simulink model "HDVSim_FCEV_Truck"  (R2019b)
settings: Solver=ode23s  StartTime=0.0  StopTime=inf  FixedStep=sample_time  MaxStep=0.1  RelTol=10e-1  EnableMultiTasking=on
model workspace (MAT data in the model file):
  Anschaffungskosten = [3707764736; 2; 1; 1; 1; 1]
  Bat.Useable_range = 0.1
  Bat.Useable_capacity = 87.5
  Bat.Type = 2
  Bat.SOC_start = 0.5
  Bat.Voltage = 800
  Bat.Efficiency_power_electronics = 0.95
  Bat.Charge_cycles = 3000
  DrvTrn = 14
  Eigenschaften = [3707764736; 2; 1; 1; 2; 2]
  Electric_Truck = 1
  Elektro_LKW = 1
  Factor_EM = 0.2
  Fahrzyklus = 2
  FuelCell.P_nom = 120
  FuelCell.NernstVoltage = [1121.27882197 1115.6396755]
  FuelCell.OperatingPoint = [171.428571429 700]
  FuelCell.MaximumPoint = [244.897959184 625.010310793]
  FuelCell.NoCells = 1015
  FuelCell.Eta = 55
  FuelCell.OperatingTemp = 65
  FuelCell.AirFlow = 7145.36039252
  FuelCell.Pressure = [1.5 1]
  FuelCell.Composition = [99.95 21 1]
  FuelCell.regV = [700 748.201780912 802.997904409 873.900542152 1076.08341487]
  FuelCell.regI = [171.428571429 128.571428571 85.7142857143 42.8571428571 2]
  FuelCell.regulation1 = [171.428571429 700]
  FuelCell.regulation2 = [128.571428571 748.201780912]
  FuelCell.regulation3 = [85.7142857143 802.997904409]
  FuelCell.regulation4 = [42.8571428571 873.900542152]
  FuelCell.regulation5 = [2 1076.08341487]
  FuelCell.Hysterese1 = [0.35 0.3]
  FuelCell.Hysterese2 = [0.4 0.35]
  FuelCell.Hysterese3 = [0.45 0.4]
  FuelCell.Hysterese4 = [0.5 0.45]
  FuelCell.Hysterese5 = [0.55 0.5]
  Fueltype = 13
  Gasart = ''
  Gewichte = [3707764736; 2; 1; 1; 3; 3]
  Hybrid_LKW = 1
  Hybrid_Truck = 1
  IZ12 = 3600
  Kosten.Kges = 1.08061767244
  Kosten.Kges_oP = 0.797760529586
  Kosten.KA = 0.104662721893
  Kosten.KA_ZM = 49440
  Kosten.KA_AN1 = 39000
  Kosten.KA_AN2 = 0
  Kosten.KA_AN3 = 0
  Kosten.tab = 6.5
  Kosten.sj = 130000
  Kosten.KK = 0.46991
  Kosten.KK_Diesel = 1.3426
  Kosten.BK_Diesel = 35
  Kosten.KK_CNG = 1.094
  Kosten.BK_CNG = 0
  Kosten.KK_LNG = 0.5671
  Kosten.BK_LNG = 0
  Kosten.IK = 100
  Kosten.KSS = 0.0046991
  Kosten.f_SS = 0.01
  Kosten.KAB = 0.0187964
  Kosten.f_AB = 0.04
  Kosten.KR = 0.0323076923077
  Kosten.KR_ZM = 400
  Kosten.BR_ZM = 6
  Kosten.KR_AN = 300
  Kosten.BR_AN = 6
  Kosten.IR = 130000
  Kosten.KW = 0.0230769230769
  Kosten.KW_ZM = 1500
  Kosten.BW = 2
  Kosten.IW = 130000
  Kosten.KV = 0.0384615384615
  Kosten.KV_ZM = 5000
  Kosten.IV = 130000
  Kosten.KM = 0.0987
  Kosten.KM_ZM = 0.141
  Kosten.f_M = 0.7
  Kosten.KS = 0.00714615384615
  Kosten.KS_ZM = 556
  Kosten.KS_AN = 373
  Kosten.IS = 130000
  Kosten.KP = 0.282857142857
  Kosten.KPSL = 19.8
  Kosten.v_avg = 70
  Kraftstoff = 13
  M_el = 1000
  Opt_groessen = []
  Parallel = 'no'
  SOC_T_EM_completely_available = 0.5
  SOC_addition = 0.4
  SOC_el = 0.8
  SOC_soll = 0.5
  SOC_target = 0.85
  Sailing_without_drag_torque = 1
  TCO = []
  TCO_Anhaenger = 1
  TCO_Trailer = 1
  T_distance_LPS_down = 250
  T_distance_LPS_up = 250
  Temp1 = [1000 1100 1200 1300 1400 1500 1600 1700 1800 1900 2000 2100 2200 2300 2400 2500 2600 2700 2800 2900 3000 3100 3200 3300 3400 3500 3600 3700 3800 3900 4000 4100 4200 4300 4400 4500 4600 4700 4800 4900 5000]
  VSim.Display = 2
  VSim.Opt = 0
  VSim.v_t = [0 0 0 0 0 0 0 0 0 0 0 0 0.1 2.22673669893 4.45336306907 5.92398131548 10.3757847916 13.2235931536 16.4384727928 19.3208442034 20.4098221432 22.5036755202 24.4402958137 25.2224117846 25.2414500209 26.5896480968 27.764074406 29.7317382984 29.2489710153 29.8958446635 31.3306398255 32.4945605422 32.2484373969 32.7472665177 34.574070202 36.2762220764 37.8657378187 37.7612251955 37.2633218769 37.960097988 39.1551886552 40.0333329677 41.4355684859 42.768336292 44.0386638817 45.25244579 46.4146842031 46.9654264036 46.4489997818 46.5754869226 47.2481025657 48.3417532766 49.3936383063 50.4067716597 51.3838125461 52.0475410751 52.7867950368 53.6833902649 54.5511282108 55.3916308392 56.206370499 56.9966877803 57.6121852605 58.3615642383 59.0897061106 59.7975811134 60.4860862919 61.1560531125 61.8082544664 62.4434104654 63.0621929387 63.6652304544 64.2531124249 64.8263914568 65.3855880065 65.9311920811 66.4636658567 66.9834460991 67.4909462072 67.9865577959 68.4706523474 68.94358275 69.4056845665 69.8572774365 70.2986658222 70.730140154 71.1519781486 71.5644446766 71.9677937776 72.3622683873 72.7481013003 73.1255161942 73.4947274795 73.8559412098 74.2093555521 74.555161059 74.8935412643 75.22467292 75.5487264183 75.86586614 76.1762506856 76.4800736479 76.7776508183 77.0691489472 77.354700525 77.6344340882 77.9084742343 78.1769412151 78.4399520593 78.6976199742 78.9500548666 79.1483067825 79.3915890977 79.6299292459 79.863425809 80.004951896 80.18236505 80.4046218047 80.6223491669 80.8356342124 81.0445617915 81.2492142762 81.4496719512 81.6460130853 81.8383140741 82.0266493037]
  Vehicle = 'FCEV'
  WPT.Voltage = 0
  WPT.P_max = 200
  WPT.expansion = 0.3
  WPT.eta = 0.92
  WPT.SOC_target = 0.8
  WPT.SOC_electric_only = 0.3
  acquisitionCosts = []
  acquisition_costs = [3707764736; 2; 1; 1; 3; 3]
  addition_for_critical_slope = 0.01
  ambient.gravity = 9.80665
  ambient.air_density = 1.1883
  ambient.friction_coeff = 0.8
  auxiliary_consumers.onBattery = 0
  auxiliary_consumers.power = 3500
  critical_altitude_difference = 50
  cycle.distance = [0 1 2 3 4 5 6 7 8 9 10 11 ... (92937 elements, 92937x1)]
  cycle.speed = [0 23.0555555556 23.0555555556 23.0555555556 23.0555555556 23.0555555556 23.0555555556 23.0555555556 23.0555555556 23.0555555556 23.0555555556 23.0555555556 ... (92937 elements, 92937x1)]
  cycle.slope = [-0.008925 -0.008925 -0.0089836957 -0.0090130435 -0.0090423913 -0.0090717391 -0.009101087 -0.0091304348 -0.0091597826 -0.0091891304 -0.0092184783 -0.0092478261 ... (92937 elements, 92937x1)]
  cycle.stop_time = [1 1 1 0 0 0 0 0 0 0 0 0 ... (92937 elements, 92937x1)]
  cycle.altitude = [0 0 0 0 0 0 0 0 0 0 0 0 ... (92937 elements, 92937x1)]
  cycle.stop_end = 15
  cycle.stop_start = 5
  cycle.speed_init = 1
  cycle.delta12 = [0 0 0 0 0 0 0 0 0 0 0 0 ... (92937 elements, 1x92937)]
  cycle.delta23 = [0 0 0 0 0 0 0 0 0 0 0 0 ... (92937 elements, 1x92937)]
  dcycle = 21
  distance_1_PPC = 175
  distance_2_PPC = 200
  distance_PPC_altitude_difference = 10000
  driver.controller_proportional_gain = 230000
  driver.controller_integral_gain = 3000
  driver.slew_rate_throttle_in = 2
  driver.slew_rate_throttle_out = -2
  driver.slew_rate_brake_in = 2
  driver.slew_rate_brake_out = -2
  em.M_max = 1600
  em.n_max = 10000
  em.n_eck = 2800
  em.P_max = 469.144502936
  em.shift_parameter.n_pref = 2632
  em.shift_parameter.n2 = 588
  em.shift_parameter.n6 = 8000
  em.shift_parameter.M_max = 1600
  em.fuel.co2_per_kwh = 478
  em.speed = [0 10 20 30 40 50 60 70 80 90 100 110 ... (1001 elements, 1x1001)]
  em.trq = [1600 1600 1600 1600 1600 1600 1600 1600 1600 1600 1600 1600 ... (1001 elements, 1x1001)]
  em.Type = 2
  em.efficiency.characteristic_map = [0.001 0.001 0.001 0.001 0.001 0.001 0.001 0.001 0.001 0.001 0.001 0.001 ... (20301 elements, 101x201)]
  em.efficiency.speed = [0 50 100 150 200 250 300 350 400 450 500 550 600 650 700 750 800 850 900 950 1000 1050 1100 1150 1200 1250 1300 1350 1400 1450 1500 1550 1600 1650 1700 1750 1800 1850 1900 1950 2000 2050 2100 2150 2200 2250 2300 2350 2400 2450 2500 2550 2600 2650 2700 2750 2800 2850 2900 2950 3000 3050 3100 3150 3200 3250 3300 3350 3400 3450 3500 3550 3600 3650 3700 3750 3800 3850 3900 3950 4000 4050 4100 4150 4200 4250 4300 4350 4400 4450 4500 4550 4600 4650 4700 4750 4800 4850 4900 4950 5000 5050 5100 5150 5200 5250 5300 5350 5400 5450 5500 5550 5600 5650 5700 5750 5800 5850 5900 5950 6000 6050 6100 6150 6200 6250 6300 6350 6400 6450 6500 6550 6600 6650 6700 6750 6800 6850 6900 6950 7000 7050 7100 7150 7200 7250 7300 7350 7400 7450 7500 7550 7600 7650 7700 7750 7800 7850 7900 7950 8000 8050 8100 8150 8200 8250 8300 8350 8400 8450 8500 8550 8600 8650 8700 8750 8800 8850 8900 8950 9000 9050 9100 9150 9200 9250 9300 9350 9400 9450 9500 9550 9600 9650 9700 9750 9800 9850 9900 9950 10000]
  em.efficiency.torque = [0 16 32 48 64 80 96 112 128 144 160 176 192 208 224 240 256 272 288 304 320 336 352 368 384 400 416 432 448 464 480 496 512 528 544 560 576 592 608 624 640 656 672 688 704 720 736 752 768 784 800 816 832 848 864 880 896 912 928 944 960 976 992 1008 1024 1040 1056 1072 1088 1104 1120 1136 1152 1168 1184 1200 1216 1232 1248 1264 1280 1296 1312 1328 1344 1360 1376 1392 1408 1424 1440 1456 1472 1488 1504 1520 1536 1552 1568 1584 1600]
  em.noEM = 2
  em_M_max = 1563
  engine.shift_parameter.n_lo = 1024
  engine.shift_parameter.n_pref = 1354
  engine.M_max = 0
  engine.number = 4
  final_drive.ratio = 10
  final_drive.trq_eff = 0.98
  ifElectric = 1
  ifOptimized = 1
  look_ahead_PPC = 250
  max_distance = 100185
  name = 'HDVSim_FCEV_Truck'
  preditiveCruiseControl = 1
  propertie = [3707764736; 2; 1; 1; 4; 4]
  prospective_cruise_control = 1
  sample_time = 0.1
  tank.v_diesel = 0
  tank.v_cng = 0
  tank.v_lng = 0
  tank.m_h2 = 20
  tires.radius = 0.4465
  tires.roll_drag_coeff.total = 0.007
  transmission.init_gear = 1
  transmission.shift_time = 1
  transmission.ratios = [3.0257 2.42471496127 1.94310164372 1.55714962713 1.24785801561 1]
  transmission.trq_eff = [0.96 0.966 0.972 0.978 0.984 0.99]
  transmission.lamda = [1.08679703675 1.05406616627 1.03254008792 1.02251301421 1.01826306417 1.0130058518]
  transmission.z = 6
  v_PPC_delta = 2.77777777778
  v_max_electrical_drive_only = 13.8888888889
  vehicle.long_truck = 0
  vehicle.payload = 19300
  vehicle.frontal_area = 10.2
  vehicle.air_drag_coeff = 0.53
  vehicle.Fx_max = 90221.18
  vehicle.F_brake_max = 309890.14
  vehicle.mass = 16370.7055705
  vehicleProperties = []
  vehicle_F_brake_max = 309890.14
  weights = []
  x = [1600 19 0.1 87.5 10 3.0257 4 0 0 1024 1354 2 2 120]
values from neighbouring files (symbols this model uses):
  FuelCell = FuelCell_design(180, 600)    [Parameterizing.m]
  transmission = Transmission_gearing(15.86, 7, 0, 0, final_drive.ratio)    [Parameterizing.m]
undefined symbols (not defined in the file or in its folder): Ion, Lithium, Vorausschauender_Tempomat
libraries: simulink x22, powerlib x4, electricdrivelib x2
(part 1 of 3)
settings Model: Creator=Alexander Suessmann  LastModifiedBy=ubuntu
settings SolverCC: ZeroCrossControl=EnableAll  AutoInsertRateTranBlk=on
settings DataIOCC: SaveFormat=Array  SignalLoggingSaveFormat=ModelDataLogs  SaveOutput=off  SignalLogging=off  DSMLogging=off  ReturnWorkspaceOutputs=off  SignalLoggingName=datalog
settings OptimizationCC: ExpressionDepthLimit=2147483647  InitFltsAndDblsToZero=on  LifeSpan=inf
settings DebuggingCC: UnderspecifiedInitializationDetection=Classic  MergeDetectMultiDrivingBlocksExec=none  SolverPrmCheckMsg=warning  MultiTaskDSMMsg=warning  MultiTaskCondExecSysMsg=none  FcnCallInpInsideContextMsg=warning  AllowSymbolicDim=off  StateNameClashWarn=warning  SFUnexpectedBacktrackingDiag=warning  SFNoUnconditionalDefaultTransitionDiag=warning  SFExecutionAtInitializationDiag=none  ArithmeticOperatorsInVariantConditions=warning
settings HardwareCC: ProdBitPerPointer=32  ProdBitPerSizeT=32  ProdBitPerPtrDiffT=32  ProdLargestAtomicFloat=None  ProdIntDivRoundTo=Undefined  ProdEndianess=Unspecified  ProdWordSize=32  ProdHWDeviceType=32-bit Generic
settings ModelReferenceCC: PropagateSignalLabelsOutOfModel=off
settings SFSimCC: SimUseLocalCustomCode=off  CustomCodeUndefinedFunction=UseInterfaceOnly
settings RTWCC: RTWUseLocalCustomCode=off
settings CodeAppCC: StateflowObjectComments=on
settings GRTTargetCC: TargetLangStandard=C89/C90 (ANSI)  CombineOutputUpdateFcns=off  GRTInterface=on
settings ConfigComp: CovMetricSettings=dw  CovSaveCumulativeToWorkspaceVar=on  CovSaveSingleToWorkspaceVar=on  CovExternalEMLEnable=off  CovSFcnEnable=off
settings SimscapeCC: SimscapeNormalizeSystem=off

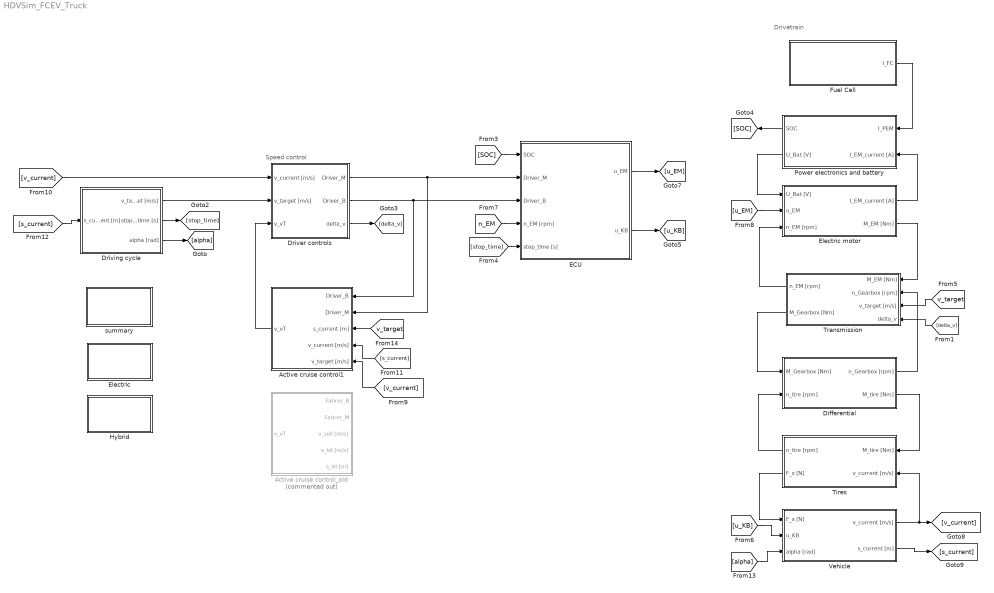
== system HDVSim_FCEV_Truck ==
  note: Speed control
  note: Drivetrain
  Active cruise control1 : Subsystem
  Active cruise control_old : Subsystem  Commented=on
  Differential : Subsystem
  Driver controls : Subsystem
  Driving cycle : Subsystem
  ECU : Subsystem
  Electric : Subsystem
  Electric motor : Subsystem
  Fuel Cell : Subsystem
  Hybrid : Subsystem
  Power electronics and battery : Subsystem
  Tires : Subsystem
  Transmission : Subsystem
  Vehicle : Subsystem
  summary : Subsystem
  wires:
  Driving cycle/1(v_target [m/s]) -> Driver controls/2(v_target [m/s])
  Driver controls/1(Driver_M) -> Active cruise control1/2(Driver_M), ECU/2(Driver_M)
  Driver controls/2(Driver_B) -> Active cruise control1/1(Driver_B), ECU/3(Driver_B)
  Driver controls/3(delta_v) -> [delta_v]
  Driving cycle/2(stop_time [s]) -> [stop_time]
  Driving cycle/3(alpha [rad]) -> [alpha]
  [v_target] -> Active cruise control1/3(s_current [m])
  [s_current] -> Active cruise control1/4(v_current [m/s])
  [v_current] -> Active cruise control1/5(v_target [m/s])
  Active cruise control1/1(v_vT) -> Driver controls/3(v_vT)
  [v_current] -> Driver controls/1(v_current [m/s])
  [s_current] -> Driving cycle/1(s_current [m])
  [SOC] -> ECU/1(SOC)
  [n_EM] -> ECU/4(n_EM [rpm])
  [stop_time] -> ECU/5(stop_time [s])
  ECU/1(u_EM) -> [u_EM]
  ECU/2(u_KB) -> [u_KB]
  Power electronics and battery/2(U_Bat [V]) -> Electric motor/1(U_Bat [V])
  Transmission/1(n_EM [rpm]) -> Electric motor/3(n_EM [rpm])
  Transmission/2(M_Gearbox [Nm]) -> Differential/1(M_Gearbox [Nm])
  Tires/1(n_tire [rpm]) -> Differential/2(n_tire [rpm])
  Tires/2(F_x [N]) -> Vehicle/1(F_x [N])
  Electric motor/1(I_EM_current [A]) -> Power electronics and battery/2(I_EM_current [A])
  Electric motor/2(M_EM [Nm]) -> Transmission/1(M_EM [Nm])
  Differential/1(n_Gearbox [rpm]) -> Transmission/2(n_Gearbox [rpm])
  Differential/2(M_tire [Nm]) -> Tires/1(M_tire [Nm])
  Vehicle/1(v_current [m/s]) -> [v_current], Tires/2(v_current [m/s])
  [u_KB] -> Vehicle/2(u_KB)
  [alpha] -> Vehicle/3(alpha [rad])
  Vehicle/2(s_current [m]) -> [s_current]
  [v_target] -> Transmission/3(v_target [m/s])
  [delta_v] -> Transmission/4(delta_v)
  [u_EM] -> Electric motor/2(u_EM)
  Power electronics and battery/1(SOC) -> [SOC]
  Fuel Cell/1(I_FC) -> Power electronics and battery/1(I_PEM)

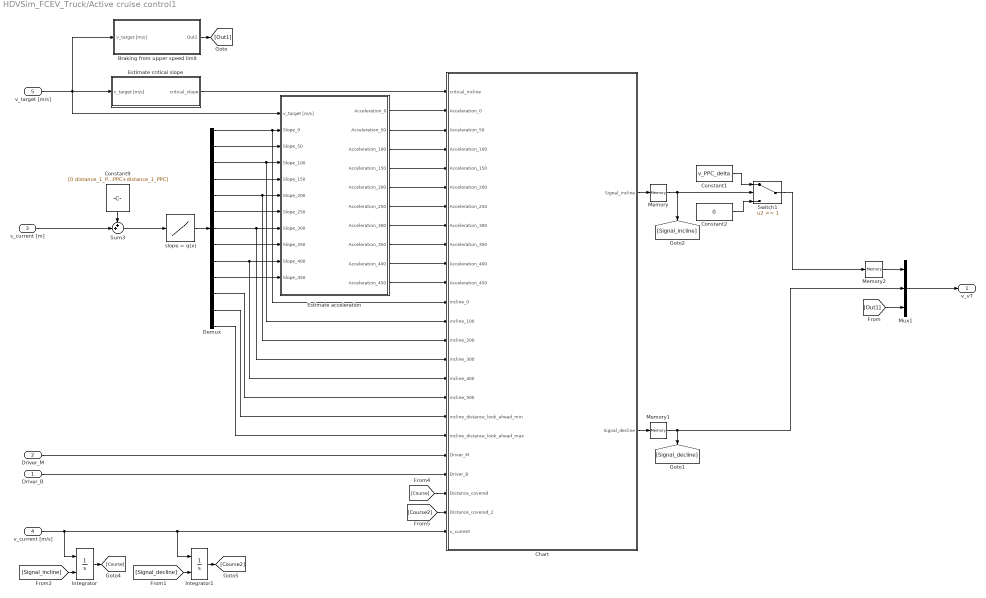
== system HDVSim_FCEV_Truck/Active cruise control1 ==
  Driver_B : Inport
  Driver_M : Inport  Port=2
  s_current [m] : Inport  Port=3
  v_current [m/s] : Inport  Port=4
  v_target [m/s] : Inport  Port=5
  Braking from upper speed limit : Subsystem
  Chart : Stateflow chart  TreatAsAtomicUnit=on
  Constant1 : Constant  Value=v_PPC_delta
  Constant2 : Constant  Value=0
  Constant9 : Constant  Value=[0 distance_1_PPC/5 2*distance_1_PPC/5 3*distance_1_PPC/5 4*distance_1_PPC/5 distance_1_PPC 6*distance_1_PPC/5 7*distance_1_PPC/5 8*distance_1_PPC/5 9*distance_1_PPC/5 10*distance_1_PPC/5 look_ahead_PPC look_ahead_PPC+distance_1_PPC]
  Demux : Demux  Outputs=13
  Estimate acceleration : Subsystem
  Estimate critical slope : Subsystem
  Integrator : Integrator  ExternalReset=rising
  Integrator1 : Integrator  ExternalReset=rising
  Memory : Memory
  Memory1 : Memory
  Memory2 : Memory
  Mux1 : Mux  Inputs=3
  Sum3 : Sum  Inputs=++|
  Switch1 : Switch  Threshold=1
  slope = q(x) : Lookup  InputValues=cycle.distance  Table=cycle.slope  LookUpMeth=Interpolation-Use End Values
  v_vT : Outport  VectorParamsAs1DForOutWhenUnconnected=off
  wires:
  slope = q(x)/1 -> Demux/1
  Demux/1 -> Chart/12(incline_0), Estimate acceleration/2(Slope_0)
  Estimate critical slope/1(critical_slope) -> Chart/1(critical_incline)
  Chart/1(Signal_incline) -> Memory/1
  v_current [m/s]/1 -> Integrator/1, Chart/24(v_current), Integrator1/1
  Memory/1 -> [Signal_incline], Switch1/2
  Mux1/1 -> v_vT/1
  Constant9/1 -> Sum3/1
  Sum3/1 -> slope = q(x)/1
  Estimate acceleration/1(Acceleration_0) -> Chart/2(Acceleration_0)
  Estimate acceleration/2(Acceleration_50) -> Chart/3(Acceleration_50)
  Estimate acceleration/3(Acceleration_100) -> Chart/4(Acceleration_100)
  Estimate acceleration/4(Acceleration_150) -> Chart/5(Acceleration_150)
  Estimate acceleration/5(Acceleration_200) -> Chart/6(Acceleration_200)
  Estimate acceleration/6(Acceleration_250) -> Chart/7(Acceleration_250)
  Estimate acceleration/7(Acceleration_300) -> Chart/8(Acceleration_300)
  Estimate acceleration/8(Acceleration_350) -> Chart/9(Acceleration_350)
  Chart/2(Signal_decline) -> Memory1/1
  Memory1/1 -> Mux1/2, [Signal_decline]
  Driver_B/1 -> Chart/21(Driver_B)
  Estimate acceleration/9(Acceleration_400) -> Chart/10(Acceleration_400)
  Estimate acceleration/10(Acceleration_450) -> Chart/11(Acceleration_450)
  Driver_M/1 -> Chart/20(Driver_M)
  Demux/2 -> Estimate acceleration/3(Slope_50)
  Demux/3 -> Chart/13(incline_100), Estimate acceleration/4(Slope_100)
  Demux/4 -> Estimate acceleration/5(Slope_150)
  Demux/5 -> Chart/14(incline_200), Estimate acceleration/6(Slope_200)
  Demux/6 -> Estimate acceleration/7("Slope_250 ")
  Demux/7 -> Chart/15(incline_300), Estimate acceleration/8(Slope_300)
  Demux/8 -> Estimate acceleration/9(Slope_350)
  Demux/9 -> Chart/16(incline_400), Estimate acceleration/10(Slope_400)
  Demux/10 -> Estimate acceleration/11(Slope_450)
  Demux/12 -> Chart/18(incline_distance_look_ahead_min)
  Demux/13 -> Chart/19(incline_distance_look_ahead_max)
  v_target [m/s]/1 -> Braking from upper speed limit/1(v_target [m/s]), Estimate acceleration/1(v_target [m/s]), Estimate critical slope/1(v_target [m/s])
  Memory2/1 -> Mux1/1
  Constant1/1 -> Switch1/1
  Constant2/1 -> Switch1/3
  Demux/11 -> Chart/17(incline_500)
  Switch1/1 -> Memory2/1
  s_current [m]/1 -> Sum3/2
  [Signal_decline] -> Integrator1/2
  Integrator1/1 -> [Course2]
  Integrator/1 -> [Course]
  [Signal_incline] -> Integrator/2
  [Course] -> Chart/22(Distance_covered)
  [Course2] -> Chart/23(Distance_covered_2)
  Braking from upper speed limit/1(Out1) -> [Out1]
  [Out1] -> Mux1/3

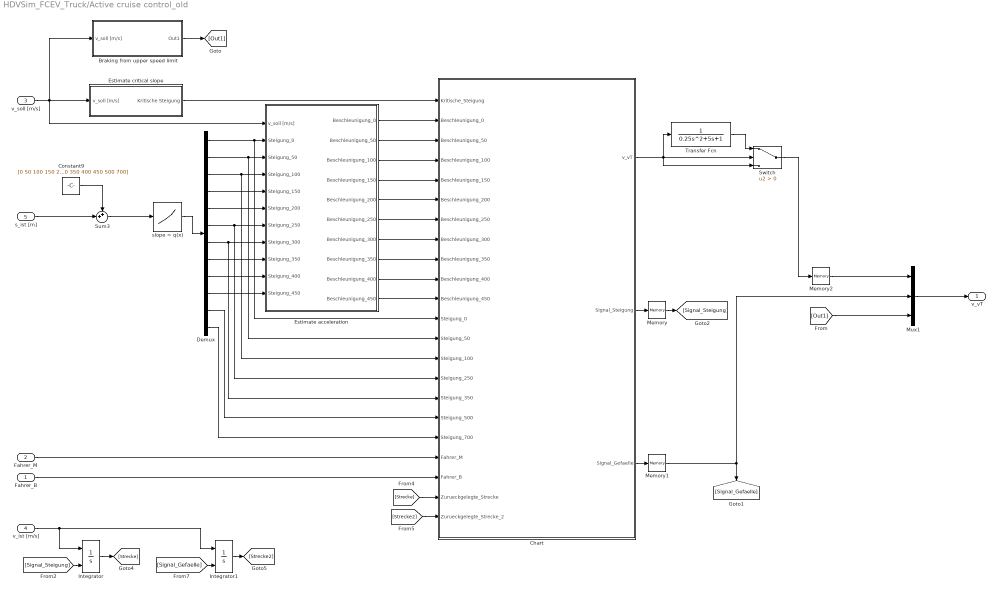
== system HDVSim_FCEV_Truck/Active cruise control_old ==
  Fahrer_B : Inport
  Fahrer_M : Inport  Port=2
  v_soll [m/s] : Inport  Port=3
  v_ist [m/s] : Inport  Port=4
  s_ist [m] : Inport  Port=5
  Braking from upper speed limit : Subsystem
  Chart : Stateflow chart  TreatAsAtomicUnit=on
  Constant9 : Constant  Value=[0 50 100 150 200 250 300 350 400 450 500 700]
  Demux : Demux  Outputs=12
  Estimate acceleration : Subsystem
  Estimate critical slope : Subsystem
  Integrator : Integrator  ExternalReset=rising
  Integrator1 : Integrator  ExternalReset=rising
  Memory : Memory
  Memory1 : Memory
  Memory2 : Memory
  Mux1 : Mux  Inputs=3
  Sum3 : Sum  Inputs=++|
  Switch : Switch  Criteria=u2 > Threshold
  Transfer Fcn : TransferFcn  Denominator=[0.25 5 1]
  slope = q(x) : Lookup  InputValues=cycle.distance  Table=cycle.slope  LookUpMeth=Interpolation-Use End Values
  v_vT : Outport  VectorParamsAs1DForOutWhenUnconnected=off
  wires:
  slope = q(x)/1 -> Demux/1
  Demux/1 -> Estimate acceleration/2(Steigung_0), Chart/12(Steigung_0)
  Estimate critical slope/1(Kritische Steigung) -> Chart/1(Kritische_Steigung)
  Chart/2(Signal_Steigung) -> Memory/1
  v_ist [m/s]/1 -> Integrator1/1, Integrator/1
  Mux1/1 -> v_vT/1
  Constant9/1 -> Sum3/1
  s_ist [m]/1 -> Sum3/2
  Sum3/1 -> slope = q(x)/1
  Estimate acceleration/1(Beschleunigung_0) -> Chart/2(Beschleunigung_0)
  Estimate acceleration/2(Beschleunigung_50) -> Chart/3(Beschleunigung_50)
  Estimate acceleration/3(Beschleunigung_100) -> Chart/4(Beschleunigung_100)
  Estimate acceleration/4(Beschleunigung_150) -> Chart/5(Beschleunigung_150)
  Estimate acceleration/5(Beschleunigung_200) -> Chart/6(Beschleunigung_200)
  Estimate acceleration/6(Beschleunigung_250) -> Chart/7(Beschleunigung_250)
  Estimate acceleration/7(Beschleunigung_300) -> Chart/8(Beschleunigung_300)
  Estimate acceleration/8(Beschleunigung_350) -> Chart/9(Beschleunigung_350)
  Chart/3(Signal_Gefaelle) -> Memory1/1
  Memory1/1 -> Mux1/2, [Signal_Gefaelle]
  Fahrer_B/1 -> Chart/20(Fahrer_B)
  Estimate acceleration/9(Beschleunigung_400) -> Chart/10(Beschleunigung_400)
  Estimate acceleration/10(Beschleunigung_450) -> Chart/11(Beschleunigung_450)
  Fahrer_M/1 -> Chart/19(Fahrer_M)
  Demux/2 -> Estimate acceleration/3(Steigung_50), Chart/13(Steigung_50)
  Demux/3 -> Estimate acceleration/4(Steigung_100), Chart/14(Steigung_100)
  Demux/4 -> Estimate acceleration/5(Steigung_150)
  Demux/5 -> Estimate acceleration/6(Steigung_200)
  Demux/6 -> Estimate acceleration/7("Steigung_250 "), Chart/15(Steigung_250)
  Demux/7 -> Estimate acceleration/8(Steigung_300), Chart/16(Steigung_350)
  Demux/8 -> Estimate acceleration/9(Steigung_350)
  Demux/9 -> Estimate acceleration/10(Steigung_400)
  Demux/10 -> Estimate acceleration/11(Steigung_450)
  Demux/11 -> Chart/17(Steigung_500)
  Demux/12 -> Chart/18(Steigung_700)
  v_soll [m/s]/1 -> Braking from upper speed limit/1(v_soll [m/s]), Estimate acceleration/1(v_soll [m/s]), Estimate critical slope/1(v_soll [m/s])
  Memory2/1 -> Mux1/1
  Switch/1 -> Memory2/1
  Chart/1(v_vT) -> Switch/2, Transfer Fcn/1, Switch/3
  Transfer Fcn/1 -> Switch/1
  [Out1] -> Mux1/3
  Braking from upper speed limit/1(Out1) -> [Out1]
  [Signal_Gefaelle] -> Integrator1/2
  [Signal_Steigung] -> Integrator/2
  Integrator/1 -> [Strecke]
  Integrator1/1 -> [Strecke2]
  [Strecke] -> Chart/21(Zurueckgelegte_Strecke)
  [Strecke2] -> Chart/22(Zurueckgelegte_Strecke_2)
  Memory/1 -> [Signal_Steigung]

== system HDVSim_FCEV_Truck/Differential ==
  note: Effective wheel torque
  M_Gearbox [Nm] : Inport
  n_tire [rpm] : Inport  Port=2
  Differential efficiency : Constant  Value=final_drive.trq_eff
  Differential gear ratio : Constant  Value=final_drive.ratio
  M' = M*i*eta : Product  Inputs=***
  Product : Product  Inputs=/  RndMeth=Zero
  Switch : Switch  ZeroCross=off
  n' *i = n : Product  RndMeth=Zero
  n_Gearbox [rpm] : Outport  VectorParamsAs1DForOutWhenUnconnected=off
  M_tire [Nm] : Outport  Port=2  VectorParamsAs1DForOutWhenUnconnected=off
  tag scope: Eta_axle_drive=global
  wires:
  M_Gearbox [Nm]/1 -> M' = M*i*eta/1, Switch/2
  n_tire [rpm]/1 -> n' *i = n/2
  Differential gear ratio/1 -> n' *i = n/1, M' = M*i*eta/3
  n' *i = n/1 -> n_Gearbox [rpm]/1
  M' = M*i*eta/1 -> M_tire [Nm]/1
  Differential efficiency/1 -> Product/1, Switch/1
  Product/1 -> Switch/3
  Switch/1 -> [Eta_axle_drive], M' = M*i*eta/2

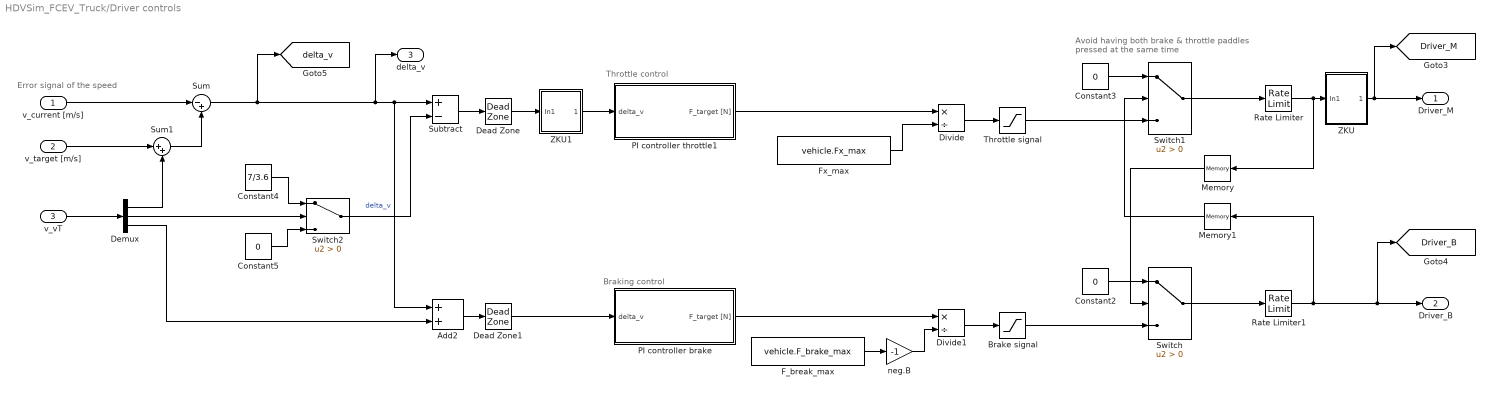
== system HDVSim_FCEV_Truck/Driver controls ==
  note: Avoid having both brake & throttle
      | paddles pressed at the same time
  note: Throttle control
  note: Braking control
  note: Error signal of the speed
  v_current [m/s] : Inport
  v_target [m/s] : Inport  Port=2
  v_vT : Inport  Port=3
  Add2 : Sum
  Brake signal : Saturate  UpperLimit=1  LowerLimit=0  ZeroCross=off
  Constant2 : Constant  Value=0
  Constant3 : Constant  Value=0
  Constant4 : Constant  Value=7/3.6
  Constant5 : Constant  Value=0
  Dead Zone : DeadZone  LowerValue=-0.1/3.6  UpperValue=0.1/3.6  ZeroCross=off  SaturateOnIntegerOverflow=on
  Dead Zone1 : DeadZone  LowerValue=-0.1/3.6  UpperValue=0  ZeroCross=off  SaturateOnIntegerOverflow=on
  Demux : Demux  Outputs=3
  Divide : Product  Inputs=*/
  Divide1 : Product  Inputs=*/
  F_break_max : Constant  Value=vehicle.F_brake_max
  Fx_max : Constant  Value=vehicle.Fx_max
  Memory : Memory
  Memory1 : Memory
  PI controller brake : Subsystem
  PI controller throttle1 : Subsystem
  Rate Limiter : RateLimiter  RisingSlewLimit=driver.slew_rate_throttle_in  FallingSlewLimit=driver.slew_rate_throttle_out  SampleTimeMode=inherited
  Rate Limiter1 : RateLimiter  RisingSlewLimit=driver.slew_rate_brake_in*100  FallingSlewLimit=driver.slew_rate_brake_out*100  SampleTimeMode=inherited
  Subtract : Sum  Inputs=+-
  Sum : Sum  Inputs=|-+
  Sum1 : Sum  Inputs=|++
  Switch : Switch  Criteria=u2 > Threshold
  Switch1 : Switch  Criteria=u2 > Threshold
  Switch2 : Switch  Criteria=u2 > Threshold
  Throttle signal : Saturate  UpperLimit=1  LowerLimit=0  ZeroCross=off
  ZKU : Subsystem
  ZKU1 : Subsystem
  neg.B : Gain  Gain=-1
  Driver_M : Outport  VectorParamsAs1DForOutWhenUnconnected=off
  Driver_B : Outport  Port=2  VectorParamsAs1DForOutWhenUnconnected=off
  delta_v : Outport  Port=3  VectorParamsAs1DForOutWhenUnconnected=off
  tag scope: Driver_B=global, Driver_M=global, delta_v=global
  wires:
  Fx_max/1 -> Divide/2
  neg.B/1 -> Divide1/2
  Divide1/1 -> Brake signal/1
  Divide/1 -> Throttle signal/1
  F_break_max/1 -> neg.B/1
  Switch/1 -> Rate Limiter1/1
  Brake signal/1 -> Switch/3
  Constant2/1 -> Switch/1
  Switch1/1 -> Rate Limiter/1
  Throttle signal/1 -> Switch1/3
  Rate Limiter1/1 -> Memory1/1, [Driver_B], Driver_B/1
  Rate Limiter/1 -> ZKU/1(In1), Memory/1
  Constant3/1 -> Switch1/1
  PI controller throttle1/1(F_target [N]) -> Divide/1
  PI controller brake/1(F_target [N]) -> Divide1/1
  ZKU/1(Out1) -> [Driver_M], Driver_M/1
  Memory/1 -> Switch/2
  Memory1/1 -> Switch1/2
  Sum1/1 -> Sum/2
  v_current [m/s]/1 -> Sum/1
  Sum/1 -> [delta_v], delta_v/1, Add2/1, Subtract/1  "delta_v"
  Dead Zone/1 -> ZKU1/1(In1)
  Dead Zone1/1 -> PI controller brake/1(delta_v)
  ZKU1/1(Out1) -> PI controller throttle1/1(delta_v)
  v_vT/1 -> Demux/1
  Switch2/1 -> Subtract/2
  Demux/2 -> Switch2/2
  Constant4/1 -> Switch2/1
  Constant5/1 -> Switch2/3
  Subtract/1 -> Dead Zone/1
  Demux/1 -> Sum1/2
  v_target [m/s]/1 -> Sum1/1
  Demux/3 -> Add2/2
  Add2/1 -> Dead Zone1/1

== system HDVSim_FCEV_Truck/Driving cycle ==
  note: Lookup tables
  s_current [m] : Inport
  Clock : Clock
  Compare To Constant : simulink/Logic and Bit Operations/Compare To Constant  relop=>=  const=max_distance  OutDataTypeStr=boolean
  Constant : Constant  Value=0
  Holding time at the beginning : Subsystem
  Holding time at the end : Subsystem
  Significant speed deviation : Subsystem
  Stop Simulation : Stop
  Stop Simulation 2 : Stop
  Switch : Switch  Criteria=u2 > Threshold
  Terminator : Terminator
  altitude : Lookup_n-D  NumberOfTableDimensions=1  BreakpointsForDimension1=cycle.distance  Table=cycle.altitude  InterpMethod=Flat  RndMeth=Simplest  InputSameDT=on
  slope : Lookup_n-D  NumberOfTableDimensions=1  BreakpointsForDimension1=cycle.distance  Table=cycle.slope  InterpMethod=Flat  RndMeth=Simplest  InputSameDT=on
  slope-> angle : Trigonometry  Operator=atan
  speed : Lookup_n-D  NumberOfTableDimensions=1  BreakpointsForDimension1=cycle.distance  Table=cycle.speed  InterpMethod=Flat  RndMeth=Simplest  InputSameDT=on
  stop_time : Lookup_n-D  NumberOfTableDimensions=1  BreakpointsForDimension1=cycle.distance  Table=cycle.stop_time  InterpMethod=Flat  RndMeth=Simplest  InputSameDT=on
  v_target [m/s] : Outport  VectorParamsAs1DForOutWhenUnconnected=off
  stop_time [s] : Outport  Port=2  VectorParamsAs1DForOutWhenUnconnected=off
  alpha [rad] : Outport  Port=3  VectorParamsAs1DForOutWhenUnconnected=off
  tag scope: altitude=global, slope_q=global, v_target=global
  wires:
  slope/1 -> slope-> angle/1, [slope_q]
  slope-> angle/1 -> alpha [rad]/1
  Compare To Constant/1 -> Holding time at the end/1(In1)
  Clock/1 -> Terminator/1  "duration"
  Switch/1 -> [v_target], v_target [m/s]/1
  s_current [m]/1 -> slope/1, Significant speed deviation/1(In1), Compare To Constant/1, altitude/1, speed/1, stop_time/1
  stop_time/1 -> stop_time [s]/1, Switch/2
  speed/1 -> Holding time at the beginning/1(In1)
  Holding time at the end/1(Out1) -> Stop Simulation/1
  Holding time at the beginning/1(Out1) -> Switch/3
  Constant/1 -> Switch/1
  altitude/1 -> [altitude]
  Significant speed deviation/1(Out1) -> Stop Simulation 2/1

== system HDVSim_FCEV_Truck/ECU ==
  SOC : Inport
  Driver_M : Inport  Port=2
  Driver_B : Inport  Port=3
  n_EM [rpm] : Inport  Port=4
  stop_time [s] : Inport  Port=5
  Approach to stop : Subsystem
  Calculations for braking : Subsystem
  Calculations for throttle : Subsystem
  Chart : Stateflow chart  TreatAsAtomicUnit=on
  Saturation : Saturate  UpperLimit=1  LowerLimit=-1
  Saturation1 : Saturate  UpperLimit=vehicle.Fx_max  LowerLimit=-vehicle.Fx_max
  Saturation2 : Saturate  UpperLimit=1  LowerLimit=0
  Scope : Scope  NumInputPorts=6
  Sum : Sum  Inputs=++|
  Sum1 : Sum  Inputs=++|
  u_EM : Outport  VectorParamsAs1DForOutWhenUnconnected=off
  u_KB : Outport  Port=2  VectorParamsAs1DForOutWhenUnconnected=off
  tag scope: REKUP=global
  wires:
  Chart/1(u_EM) -> Saturation/1
  Chart/2(u_KB) -> Saturation2/1
  Sum1/1 -> Calculations for braking/2(Driver_B), Chart/2(Driver_B)
  Sum/1 -> Calculations for throttle/1(Driver_M), Chart/1(Driver_M)
  Calculations for braking/2(F_brake_elec_max [N]) -> Saturation1/1
  Calculations for braking/1(F_brake_required [N]) -> Chart/6(F_brake_required)
  SOC/1 -> Chart/3(SOC), Calculations for throttle/2(SOC)
  Calculations for throttle/2(M_Required [Nm]) -> Chart/5(M_required)
  Saturation2/1 -> Scope/2, u_KB/1  "u_KB"
  Chart/3(ROLL) -> Scope/3  "ROLL"
  Chart/4(REKUP) -> [REKUP], Scope/4  "REKUP"
  [slope_q] -> Scope/5  "Slope"
  Calculations for throttle/1(M_EM_max [Nm]) -> Calculations for braking/1(M_EM_max [Nm]), Chart/4(M_EM_max)
  [SOC] -> Scope/6  "SOC"
  Saturation/1 -> u_EM/1, Scope/1  "u_EM"
  stop_time [s]/1 -> Approach to stop/1(stop_time [s])
  Approach to stop/2(Driver_B) -> Sum1/1
  Approach to stop/1(Driver_M) -> Sum/1
  Driver_M/1 -> Sum/2
  Driver_B/1 -> Sum1/2
  n_EM [rpm]/1 -> Calculations for throttle/3(n_EM [rpm])
  [gear] -> Calculations for braking/3(Gear)
  Saturation1/1 -> Chart/7(F_brake_el_max)
